simulink model "VirtualADAPT"  (Simulink 8.9)
settings: Solver=ode45  StartTime=0.0  StopTime=inf  RelTol=1e-3  EnableMultiTasking=on
PostLoadFcn:
  | FaultInjectionGUIHandle = FaultInjectionGUI;
InitFcn:
  | VirtualADAPTDataStructure
undefined symbols (not defined in the file or in its folder): ln
libraries: simulink x363
(part 26 of 26)

== system VirtualADAPT/VirtualADAPTv1/PowerDistribution/ACLoadBank1/L1A/LGT6/LightBulb1/TemperatureModel/Rtheta/Computing R ==
  In1 : Inport
  RNominal : Inport  Port=2
  Fault Profile : Inport  Port=3
  Step : Inport  Port=4
  Ramp : Inport  Port=5
  JuncDB : Inport  Port=6
  Bond : Inport  Port=7
  Junction Type : Inport  Port=8
  CalcCausality : Fcn  Expr=(u(1) == u(2) && u(3) ==1) || (u(1) !=u(2) && u(3) == 0)
  CalcOutput : Fcn  Expr=(u(1)*u(2)^u(3))
  MinusOne : Constant  Value=-1
  Mux1 : Mux  Inputs=3
  Mux2 : Mux  Inputs=3
  One : Constant
  Switch1 : MultiPortSwitch  SaturateOnIntegerOverflow=on  InputSameDT=on
  Switch2 : MultiPortSwitch  DataPortOrder=Zero-based contiguous  Inputs=2  zeroidx=on  SaturateOnIntegerOverflow=on  InputSameDT=on
  Out1 : Outport
  Out2 : Outport  Port=2
  wires:
  In1/1 -> Mux2/1
  Fault Profile/1 -> Switch1/1
  RNominal/1 -> Switch1/2
  Step/1 -> Switch1/3
  Ramp/1 -> Switch1/4
  Switch1/1 -> Out2/1, Mux2/2
  JuncDB/1 -> Mux1/1
  Bond/1 -> Mux1/2
  Junction Type/1 -> Mux1/3
  Mux1/1 -> CalcCausality/1
  CalcCausality/1 -> Switch2/1
  One/1 -> Switch2/2
  MinusOne/1 -> Switch2/3
  Switch2/1 -> Mux2/3
  Mux2/1 -> CalcOutput/1
  CalcOutput/1 -> Out1/1

== system VirtualADAPT/VirtualADAPTv1/PowerDistribution/ACLoadBank1/L1A/LGT6/LightBulb1/TemperatureModel/SfPs/Fault Inject ==
  Nominal : Inport
  Fault Profile : Inport  Port=2
  Step : Inport  Port=3
  Ramp : Inport  Port=4
  Bias : Inport  Port=5
  stuckAt : Inport  Port=6
  Switch1 : MultiPortSwitch  Inputs=5  SaturateOnIntegerOverflow=on  InputSameDT=on
  Out1 : Outport
  wires:
  Nominal/1 -> Switch1/2
  Fault Profile/1 -> Switch1/1
  Step/1 -> Switch1/3
  Ramp/1 -> Switch1/4
  Bias/1 -> Switch1/5
  stuckAt/1 -> Switch1/6
  Switch1/1 -> Out1/1

== system VirtualADAPT/VirtualADAPTv1/PowerDistribution/ACLoadBank1/L1A/LGT6/LightBulb2/TemperatureModel/AmbTemp/Fault Inject ==
  Nominal : Inport
  Fault Profile : Inport  Port=2
  Step : Inport  Port=3
  Ramp : Inport  Port=4
  Bias : Inport  Port=5
  stuckAt : Inport  Port=6
  Switch1 : MultiPortSwitch  Inputs=5  SaturateOnIntegerOverflow=on  InputSameDT=on
  Out1 : Outport
  wires:
  Nominal/1 -> Switch1/2
  Fault Profile/1 -> Switch1/1
  Step/1 -> Switch1/3
  Ramp/1 -> Switch1/4
  Bias/1 -> Switch1/5
  stuckAt/1 -> Switch1/6
  Switch1/1 -> Out1/1

== system VirtualADAPT/VirtualADAPTv1/PowerDistribution/ACLoadBank1/L1A/LGT6/LightBulb2/TemperatureModel/Ctheta/Fault Inject ==
  Nominal : Inport
  Fault Profile : Inport  Port=2
  Step : Inport  Port=3
  Ramp : Inport  Port=4
  Bias : Inport  Port=5
  stuckAt : Inport  Port=6
  Switch1 : MultiPortSwitch  Inputs=5  SaturateOnIntegerOverflow=on  InputSameDT=on
  Out1 : Outport
  wires:
  Nominal/1 -> Switch1/2
  Fault Profile/1 -> Switch1/1
  Step/1 -> Switch1/3
  Ramp/1 -> Switch1/4
  Bias/1 -> Switch1/5
  stuckAt/1 -> Switch1/6
  Switch1/1 -> Out1/1

== system VirtualADAPT/VirtualADAPTv1/PowerDistribution/ACLoadBank1/L1A/LGT6/LightBulb2/TemperatureModel/Rtheta/Computing R ==
  In1 : Inport
  RNominal : Inport  Port=2
  Fault Profile : Inport  Port=3
  Step : Inport  Port=4
  Ramp : Inport  Port=5
  JuncDB : Inport  Port=6
  Bond : Inport  Port=7
  Junction Type : Inport  Port=8
  CalcCausality : Fcn  Expr=(u(1) == u(2) && u(3) ==1) || (u(1) !=u(2) && u(3) == 0)
  CalcOutput : Fcn  Expr=(u(1)*u(2)^u(3))
  MinusOne : Constant  Value=-1
  Mux1 : Mux  Inputs=3
  Mux2 : Mux  Inputs=3
  One : Constant
  Switch1 : MultiPortSwitch  SaturateOnIntegerOverflow=on  InputSameDT=on
  Switch2 : MultiPortSwitch  DataPortOrder=Zero-based contiguous  Inputs=2  zeroidx=on  SaturateOnIntegerOverflow=on  InputSameDT=on
  Out1 : Outport
  Out2 : Outport  Port=2
  wires:
  In1/1 -> Mux2/1
  Fault Profile/1 -> Switch1/1
  RNominal/1 -> Switch1/2
  Step/1 -> Switch1/3
  Ramp/1 -> Switch1/4
  Switch1/1 -> Out2/1, Mux2/2
  JuncDB/1 -> Mux1/1
  Bond/1 -> Mux1/2
  Junction Type/1 -> Mux1/3
  Mux1/1 -> CalcCausality/1
  CalcCausality/1 -> Switch2/1
  One/1 -> Switch2/2
  MinusOne/1 -> Switch2/3
  Switch2/1 -> Mux2/3
  Mux2/1 -> CalcOutput/1
  CalcOutput/1 -> Out1/1

== system VirtualADAPT/VirtualADAPTv1/PowerDistribution/ACLoadBank1/L1A/LGT6/LightBulb2/TemperatureModel/SfPs/Fault Inject ==
  Nominal : Inport
  Fault Profile : Inport  Port=2
  Step : Inport  Port=3
  Ramp : Inport  Port=4
  Bias : Inport  Port=5
  stuckAt : Inport  Port=6
  Switch1 : MultiPortSwitch  Inputs=5  SaturateOnIntegerOverflow=on  InputSameDT=on
  Out1 : Outport
  wires:
  Nominal/1 -> Switch1/2
  Fault Profile/1 -> Switch1/1
  Step/1 -> Switch1/3
  Ramp/1 -> Switch1/4
  Bias/1 -> Switch1/5
  stuckAt/1 -> Switch1/6
  Switch1/1 -> Out1/1

== system VirtualADAPT/VirtualADAPTv1/PowerDistribution/ACLoadBank1/L1A/LGT6/LightBulb3/TemperatureModel/AmbTemp/Fault Inject ==
  Nominal : Inport
  Fault Profile : Inport  Port=2
  Step : Inport  Port=3
  Ramp : Inport  Port=4
  Bias : Inport  Port=5
  stuckAt : Inport  Port=6
  Switch1 : MultiPortSwitch  Inputs=5  SaturateOnIntegerOverflow=on  InputSameDT=on
  Out1 : Outport
  wires:
  Nominal/1 -> Switch1/2
  Fault Profile/1 -> Switch1/1
  Step/1 -> Switch1/3
  Ramp/1 -> Switch1/4
  Bias/1 -> Switch1/5
  stuckAt/1 -> Switch1/6
  Switch1/1 -> Out1/1

== system VirtualADAPT/VirtualADAPTv1/PowerDistribution/ACLoadBank1/L1A/LGT6/LightBulb3/TemperatureModel/Ctheta/Fault Inject ==
  Nominal : Inport
  Fault Profile : Inport  Port=2
  Step : Inport  Port=3
  Ramp : Inport  Port=4
  Bias : Inport  Port=5
  stuckAt : Inport  Port=6
  Switch1 : MultiPortSwitch  Inputs=5  SaturateOnIntegerOverflow=on  InputSameDT=on
  Out1 : Outport
  wires:
  Nominal/1 -> Switch1/2
  Fault Profile/1 -> Switch1/1
  Step/1 -> Switch1/3
  Ramp/1 -> Switch1/4
  Bias/1 -> Switch1/5
  stuckAt/1 -> Switch1/6
  Switch1/1 -> Out1/1

== system VirtualADAPT/VirtualADAPTv1/PowerDistribution/ACLoadBank1/L1A/LGT6/LightBulb3/TemperatureModel/Rtheta/Computing R ==
  In1 : Inport
  RNominal : Inport  Port=2
  Fault Profile : Inport  Port=3
  Step : Inport  Port=4
  Ramp : Inport  Port=5
  JuncDB : Inport  Port=6
  Bond : Inport  Port=7
  Junction Type : Inport  Port=8
  CalcCausality : Fcn  Expr=(u(1) == u(2) && u(3) ==1) || (u(1) !=u(2) && u(3) == 0)
  CalcOutput : Fcn  Expr=(u(1)*u(2)^u(3))
  MinusOne : Constant  Value=-1
  Mux1 : Mux  Inputs=3
  Mux2 : Mux  Inputs=3
  One : Constant
  Switch1 : MultiPortSwitch  SaturateOnIntegerOverflow=on  InputSameDT=on
  Switch2 : MultiPortSwitch  DataPortOrder=Zero-based contiguous  Inputs=2  zeroidx=on  SaturateOnIntegerOverflow=on  InputSameDT=on
  Out1 : Outport
  Out2 : Outport  Port=2
  wires:
  In1/1 -> Mux2/1
  Fault Profile/1 -> Switch1/1
  RNominal/1 -> Switch1/2
  Step/1 -> Switch1/3
  Ramp/1 -> Switch1/4
  Switch1/1 -> Out2/1, Mux2/2
  JuncDB/1 -> Mux1/1
  Bond/1 -> Mux1/2
  Junction Type/1 -> Mux1/3
  Mux1/1 -> CalcCausality/1
  CalcCausality/1 -> Switch2/1
  One/1 -> Switch2/2
  MinusOne/1 -> Switch2/3
  Switch2/1 -> Mux2/3
  Mux2/1 -> CalcOutput/1
  CalcOutput/1 -> Out1/1

== system VirtualADAPT/VirtualADAPTv1/PowerDistribution/ACLoadBank1/L1A/LGT6/LightBulb3/TemperatureModel/SfPs/Fault Inject ==
  Nominal : Inport
  Fault Profile : Inport  Port=2
  Step : Inport  Port=3
  Ramp : Inport  Port=4
  Bias : Inport  Port=5
  stuckAt : Inport  Port=6
  Switch1 : MultiPortSwitch  Inputs=5  SaturateOnIntegerOverflow=on  InputSameDT=on
  Out1 : Outport
  wires:
  Nominal/1 -> Switch1/2
  Fault Profile/1 -> Switch1/1
  Step/1 -> Switch1/3
  Ramp/1 -> Switch1/4
  Bias/1 -> Switch1/5
  stuckAt/1 -> Switch1/6
  Switch1/1 -> Out1/1

== system VirtualADAPT/VirtualADAPTv1/PowerDistribution/ACLoadBank2/L2E/LGT7/LightBulb1/TemperatureModel/AmbTemp/Fault Inject ==
  Nominal : Inport
  Fault Profile : Inport  Port=2
  Step : Inport  Port=3
  Ramp : Inport  Port=4
  Bias : Inport  Port=5
  stuckAt : Inport  Port=6
  Switch1 : MultiPortSwitch  Inputs=5  SaturateOnIntegerOverflow=on  InputSameDT=on
  Out1 : Outport
  wires:
  Nominal/1 -> Switch1/2
  Fault Profile/1 -> Switch1/1
  Step/1 -> Switch1/3
  Ramp/1 -> Switch1/4
  Bias/1 -> Switch1/5
  stuckAt/1 -> Switch1/6
  Switch1/1 -> Out1/1

== system VirtualADAPT/VirtualADAPTv1/PowerDistribution/ACLoadBank2/L2E/LGT7/LightBulb1/TemperatureModel/Ctheta/Fault Inject ==
  Nominal : Inport
  Fault Profile : Inport  Port=2
  Step : Inport  Port=3
  Ramp : Inport  Port=4
  Bias : Inport  Port=5
  stuckAt : Inport  Port=6
  Switch1 : MultiPortSwitch  Inputs=5  SaturateOnIntegerOverflow=on  InputSameDT=on
  Out1 : Outport
  wires:
  Nominal/1 -> Switch1/2
  Fault Profile/1 -> Switch1/1
  Step/1 -> Switch1/3
  Ramp/1 -> Switch1/4
  Bias/1 -> Switch1/5
  stuckAt/1 -> Switch1/6
  Switch1/1 -> Out1/1

== system VirtualADAPT/VirtualADAPTv1/PowerDistribution/ACLoadBank2/L2E/LGT7/LightBulb1/TemperatureModel/Rtheta/Computing R ==
  In1 : Inport
  RNominal : Inport  Port=2
  Fault Profile : Inport  Port=3
  Step : Inport  Port=4
  Ramp : Inport  Port=5
  JuncDB : Inport  Port=6
  Bond : Inport  Port=7
  Junction Type : Inport  Port=8
  CalcCausality : Fcn  Expr=(u(1) == u(2) && u(3) ==1) || (u(1) !=u(2) && u(3) == 0)
  CalcOutput : Fcn  Expr=(u(1)*u(2)^u(3))
  MinusOne : Constant  Value=-1
  Mux1 : Mux  Inputs=3
  Mux2 : Mux  Inputs=3
  One : Constant
  Switch1 : MultiPortSwitch  SaturateOnIntegerOverflow=on  InputSameDT=on
  Switch2 : MultiPortSwitch  DataPortOrder=Zero-based contiguous  Inputs=2  zeroidx=on  SaturateOnIntegerOverflow=on  InputSameDT=on
  Out1 : Outport
  Out2 : Outport  Port=2
  wires:
  In1/1 -> Mux2/1
  Fault Profile/1 -> Switch1/1
  RNominal/1 -> Switch1/2
  Step/1 -> Switch1/3
  Ramp/1 -> Switch1/4
  Switch1/1 -> Out2/1, Mux2/2
  JuncDB/1 -> Mux1/1
  Bond/1 -> Mux1/2
  Junction Type/1 -> Mux1/3
  Mux1/1 -> CalcCausality/1
  CalcCausality/1 -> Switch2/1
  One/1 -> Switch2/2
  MinusOne/1 -> Switch2/3
  Switch2/1 -> Mux2/3
  Mux2/1 -> CalcOutput/1
  CalcOutput/1 -> Out1/1

== system VirtualADAPT/VirtualADAPTv1/PowerDistribution/ACLoadBank2/L2E/LGT7/LightBulb1/TemperatureModel/SfPs/Fault Inject ==
  Nominal : Inport
  Fault Profile : Inport  Port=2
  Step : Inport  Port=3
  Ramp : Inport  Port=4
  Bias : Inport  Port=5
  stuckAt : Inport  Port=6
  Switch1 : MultiPortSwitch  Inputs=5  SaturateOnIntegerOverflow=on  InputSameDT=on
  Out1 : Outport
  wires:
  Nominal/1 -> Switch1/2
  Fault Profile/1 -> Switch1/1
  Step/1 -> Switch1/3
  Ramp/1 -> Switch1/4
  Bias/1 -> Switch1/5
  stuckAt/1 -> Switch1/6
  Switch1/1 -> Out1/1

== system VirtualADAPT/VirtualADAPTv1/PowerDistribution/ACLoadBank2/L2E/LGT7/LightBulb2/TemperatureModel/AmbTemp/Fault Inject ==
  Nominal : Inport
  Fault Profile : Inport  Port=2
  Step : Inport  Port=3
  Ramp : Inport  Port=4
  Bias : Inport  Port=5
  stuckAt : Inport  Port=6
  Switch1 : MultiPortSwitch  Inputs=5  SaturateOnIntegerOverflow=on  InputSameDT=on
  Out1 : Outport
  wires:
  Nominal/1 -> Switch1/2
  Fault Profile/1 -> Switch1/1
  Step/1 -> Switch1/3
  Ramp/1 -> Switch1/4
  Bias/1 -> Switch1/5
  stuckAt/1 -> Switch1/6
  Switch1/1 -> Out1/1

== system VirtualADAPT/VirtualADAPTv1/PowerDistribution/ACLoadBank2/L2E/LGT7/LightBulb2/TemperatureModel/Ctheta/Fault Inject ==
  Nominal : Inport
  Fault Profile : Inport  Port=2
  Step : Inport  Port=3
  Ramp : Inport  Port=4
  Bias : Inport  Port=5
  stuckAt : Inport  Port=6
  Switch1 : MultiPortSwitch  Inputs=5  SaturateOnIntegerOverflow=on  InputSameDT=on
  Out1 : Outport
  wires:
  Nominal/1 -> Switch1/2
  Fault Profile/1 -> Switch1/1
  Step/1 -> Switch1/3
  Ramp/1 -> Switch1/4
  Bias/1 -> Switch1/5
  stuckAt/1 -> Switch1/6
  Switch1/1 -> Out1/1

== system VirtualADAPT/VirtualADAPTv1/PowerDistribution/ACLoadBank2/L2E/LGT7/LightBulb2/TemperatureModel/Rtheta/Computing R ==
  In1 : Inport
  RNominal : Inport  Port=2
  Fault Profile : Inport  Port=3
  Step : Inport  Port=4
  Ramp : Inport  Port=5
  JuncDB : Inport  Port=6
  Bond : Inport  Port=7
  Junction Type : Inport  Port=8
  CalcCausality : Fcn  Expr=(u(1) == u(2) && u(3) ==1) || (u(1) !=u(2) && u(3) == 0)
  CalcOutput : Fcn  Expr=(u(1)*u(2)^u(3))
  MinusOne : Constant  Value=-1
  Mux1 : Mux  Inputs=3
  Mux2 : Mux  Inputs=3
  One : Constant
  Switch1 : MultiPortSwitch  SaturateOnIntegerOverflow=on  InputSameDT=on
  Switch2 : MultiPortSwitch  DataPortOrder=Zero-based contiguous  Inputs=2  zeroidx=on  SaturateOnIntegerOverflow=on  InputSameDT=on
  Out1 : Outport
  Out2 : Outport  Port=2
  wires:
  In1/1 -> Mux2/1
  Fault Profile/1 -> Switch1/1
  RNominal/1 -> Switch1/2
  Step/1 -> Switch1/3
  Ramp/1 -> Switch1/4
  Switch1/1 -> Out2/1, Mux2/2
  JuncDB/1 -> Mux1/1
  Bond/1 -> Mux1/2
  Junction Type/1 -> Mux1/3
  Mux1/1 -> CalcCausality/1
  CalcCausality/1 -> Switch2/1
  One/1 -> Switch2/2
  MinusOne/1 -> Switch2/3
  Switch2/1 -> Mux2/3
  Mux2/1 -> CalcOutput/1
  CalcOutput/1 -> Out1/1

== system VirtualADAPT/VirtualADAPTv1/PowerDistribution/ACLoadBank2/L2E/LGT7/LightBulb2/TemperatureModel/SfPs/Fault Inject ==
  Nominal : Inport
  Fault Profile : Inport  Port=2
  Step : Inport  Port=3
  Ramp : Inport  Port=4
  Bias : Inport  Port=5
  stuckAt : Inport  Port=6
  Switch1 : MultiPortSwitch  Inputs=5  SaturateOnIntegerOverflow=on  InputSameDT=on
  Out1 : Outport
  wires:
  Nominal/1 -> Switch1/2
  Fault Profile/1 -> Switch1/1
  Step/1 -> Switch1/3
  Ramp/1 -> Switch1/4
  Bias/1 -> Switch1/5
  stuckAt/1 -> Switch1/6
  Switch1/1 -> Out1/1

== system VirtualADAPT/VirtualADAPTv1/PowerDistribution/ACLoadBank2/L2E/LGT7/LightBulb3/TemperatureModel/AmbTemp/Fault Inject ==
  Nominal : Inport
  Fault Profile : Inport  Port=2
  Step : Inport  Port=3
  Ramp : Inport  Port=4
  Bias : Inport  Port=5
  stuckAt : Inport  Port=6
  Switch1 : MultiPortSwitch  Inputs=5  SaturateOnIntegerOverflow=on  InputSameDT=on
  Out1 : Outport
  wires:
  Nominal/1 -> Switch1/2
  Fault Profile/1 -> Switch1/1
  Step/1 -> Switch1/3
  Ramp/1 -> Switch1/4
  Bias/1 -> Switch1/5
  stuckAt/1 -> Switch1/6
  Switch1/1 -> Out1/1

== system VirtualADAPT/VirtualADAPTv1/PowerDistribution/ACLoadBank2/L2E/LGT7/LightBulb3/TemperatureModel/Ctheta/Fault Inject ==
  Nominal : Inport
  Fault Profile : Inport  Port=2
  Step : Inport  Port=3
  Ramp : Inport  Port=4
  Bias : Inport  Port=5
  stuckAt : Inport  Port=6
  Switch1 : MultiPortSwitch  Inputs=5  SaturateOnIntegerOverflow=on  InputSameDT=on
  Out1 : Outport
  wires:
  Nominal/1 -> Switch1/2
  Fault Profile/1 -> Switch1/1
  Step/1 -> Switch1/3
  Ramp/1 -> Switch1/4
  Bias/1 -> Switch1/5
  stuckAt/1 -> Switch1/6
  Switch1/1 -> Out1/1

== system VirtualADAPT/VirtualADAPTv1/PowerDistribution/ACLoadBank2/L2E/LGT7/LightBulb3/TemperatureModel/Rtheta/Computing R ==
  In1 : Inport
  RNominal : Inport  Port=2
  Fault Profile : Inport  Port=3
  Step : Inport  Port=4
  Ramp : Inport  Port=5
  JuncDB : Inport  Port=6
  Bond : Inport  Port=7
  Junction Type : Inport  Port=8
  CalcCausality : Fcn  Expr=(u(1) == u(2) && u(3) ==1) || (u(1) !=u(2) && u(3) == 0)
  CalcOutput : Fcn  Expr=(u(1)*u(2)^u(3))
  MinusOne : Constant  Value=-1
  Mux1 : Mux  Inputs=3
  Mux2 : Mux  Inputs=3
  One : Constant
  Switch1 : MultiPortSwitch  SaturateOnIntegerOverflow=on  InputSameDT=on
  Switch2 : MultiPortSwitch  DataPortOrder=Zero-based contiguous  Inputs=2  zeroidx=on  SaturateOnIntegerOverflow=on  InputSameDT=on
  Out1 : Outport
  Out2 : Outport  Port=2
  wires:
  In1/1 -> Mux2/1
  Fault Profile/1 -> Switch1/1
  RNominal/1 -> Switch1/2
  Step/1 -> Switch1/3
  Ramp/1 -> Switch1/4
  Switch1/1 -> Out2/1, Mux2/2
  JuncDB/1 -> Mux1/1
  Bond/1 -> Mux1/2
  Junction Type/1 -> Mux1/3
  Mux1/1 -> CalcCausality/1
  CalcCausality/1 -> Switch2/1
  One/1 -> Switch2/2
  MinusOne/1 -> Switch2/3
  Switch2/1 -> Mux2/3
  Mux2/1 -> CalcOutput/1
  CalcOutput/1 -> Out1/1

== system VirtualADAPT/VirtualADAPTv1/PowerDistribution/ACLoadBank2/L2E/LGT7/LightBulb3/TemperatureModel/SfPs/Fault Inject ==
  Nominal : Inport
  Fault Profile : Inport  Port=2
  Step : Inport  Port=3
  Ramp : Inport  Port=4
  Bias : Inport  Port=5
  stuckAt : Inport  Port=6
  Switch1 : MultiPortSwitch  Inputs=5  SaturateOnIntegerOverflow=on  InputSameDT=on
  Out1 : Outport
  wires:
  Nominal/1 -> Switch1/2
  Fault Profile/1 -> Switch1/1
  Step/1 -> Switch1/3
  Ramp/1 -> Switch1/4
  Bias/1 -> Switch1/5
  stuckAt/1 -> Switch1/6
  Switch1/1 -> Out1/1
